simulink library "ee_lib_for_power_systems"  (R2025b)
settings: Solver=VariableStepAuto  StartTime=0.0  StopTime=10.0  RelTol=1e-3
undefined symbols (not defined in the file or in its folder): Alt_PolePair_Count, C3m, DiscIntNeverNeededParam, Enum1to12, L3m, Total_SM_Inertia, Ts
libraries: fl_lib x291, nesl_utility x219, ee_lib x139, custom_lib x12, $bdroot x10, ee_sl_lib x3, eeTransforms x2, eeGeneralControl x1, simulink x1
(part 3 of 11)

== system ee_lib_for_power_systems/Power Converters ==
  note: Lowest Fidelity
      | Use if switching not required.
      | Will short circuit AC if mod is 0.
  note: Three-Phase Rectifiers
  note: Other
  note: Three-Phase Inverters (simulation performance improves and fidelity decreases as you move from right to left)
  note: Individual Switches
  note: Link to Simscape Electrical Library
  note: Copyright (C) 2025, The MathWorks, Inc.
  note: Use if switching not required.
      | Rectifier mode if open is 1.
  note: Can accept subcycle average PWM.
      | Will short circuit AC if g is 0.
  note: Can accept subcycle average PWM.
      | Rectifier mode if open is 1.
  note: Use if switching required and diode forward voltage can be neglected.
      | Rectifier mode if open is 1.
  note: Highest Fidelity
      | Use if switching required and diode forward voltage cannot be neglected.
  note: Use for fastest thyristor bridge performance.
  note: Use for fastest half-bridge bridge performance.
  " Ideal Switch Three-Level NPC" : Subsystem  mask{Vf=1e-3, Ron=1e-3, Goff=1e-6, run_time=off}
      mask prompts: Vf "Forward Voltage (Vf)"; Ron "On Resistance (Ohm)"; Goff "Off Conductance (1/Ohm)"; run_time "Set parameters to run-time (model does not recompile if parameters change value)"
      mask Callback run_time:
      | run_time = get_param(gcb,'run_time');
      | 
      | path_converter = [gcb,'/converter'];
      | 
      | switch run_time
      |     case 'off'
      |         pcr = 'compiletime';
      |     case 'on'
      |         pcr = 'runtime';
      | end
      | 
      | set_param(path_converter,'Ron_conf',pcr)
      | set_param(path_converter,'Goff_conf',pcr)
      | set_param(path_converter,'Vf_conf',pcr)
      mask DialogControl DescGroupVar Prompt: Three-Level NPC Converter
      mask Display: disp('Ideal Switch\nThree-Level\nNPC')
  Average-Value VSC (Three-Phase) : Subsystem  mask{Ploss=1, run_time=off}
      mask prompts: Ploss "Fixed Power Loss (W)"; run_time "Set parameters to run-time (model does not recompile if parameters change value)"
      settings: PortSchema="{\"entries\":[{\"content\":{\"sides\":[{\"content\":{\"connectorIds\":[\"LConn1\"]},\"type\":\"ConnectorPlacement.EquallySpacedRectSide\",\"uuid\":\"86da145b-c877-4513-b106-d0dfb0b4d5fc\"},{\"content\":{\"connectorIds\":[\"RConn1\",\"RConn2\"],\"side\":\"RIGHT\"},\"type\":\"ConnectorPlacement.EquallySpacedRectSide\",\"uuid\":\"c4ddf9e5-f742-41c1-b9ac-c2cdd1e548bc\"},{\"content\":{\"connectorIds\":[\"In1\"],\"side\":\"TOP\"},\"type\":\"ConnectorPlacement.EquallySpacedRectSide\",\"uuid\":\"dcce36a4-1529-4432-9ca7-d054bd477a1d\"}]},\"type\":\"ConnectorPlacement.EquallySpacedConnector\",\"uuid\":\"4f4158e8-7d48-4204-9748-7ea34849688b\"}],\"packageUris\":[\"http://schema.mathworks.com/mf0/Connector/R2020b\"],\"version\":\"1.0\"}"
      mask Callback run_time:
      | run_time = get_param(gcb,'run_time');
      | 
      | path_converter = [gcb,'/converter'];
      | 
      | switch run_time
      |     case 'off'
      |         pcr = 'compiletime';
      |     case 'on'
      |         pcr = 'runtime';
      | end
      | 
      | set_param(path_converter,'Pfixed_conf',pcr)
      mask DialogControl Container1 Prompt: Average-Value VSC (Three-Phase)
      mask DialogControl Container2 Prompt: Parameters
      mask Display: disp('Average-Value\nVSC Bridge')
  Diode Bridge (Three-Phase) : Subsystem  mask{Vf=0.6, Ron=1e-3, Goff=1e-5}
      mask prompts: Vf "Forward Voltage (V)"; Ron "On Resistance (Ohm)"; Goff "Off Conductance"
      mask DialogControl DescGroupVar Prompt: Diode Bridge
      mask Display: disp('Diode\nBridge')
  GTO : Subsystem  mask{Ron=1e-5, Goff=1e-5, Vf=0.6, run_time=off}
      mask prompts: Ron "Ron (Ohm)"; Goff "Goff (1/Ohm)"; Vf "Vf (V)"; run_time "Set parameters to run-time (model does not recompile if parameters change value)"
      settings: PortSchema="{\"entries\":[{\"content\":{\"sides\":[{\"content\":{\"connectorIds\":[\"LConn1\"]},\"type\":\"ConnectorPlacement.EquallySpacedRectSide\",\"uuid\":\"886964a3-25ea-479f-932a-c2787c0416aa\"},{\"content\":{\"connectorIds\":[\"RConn1\"],\"side\":\"RIGHT\"},\"type\":\"ConnectorPlacement.EquallySpacedRectSide\",\"uuid\":\"91f12607-1466-497f-b2f1-8e90b455b295\"},{\"content\":{\"connectorIds\":[\"In1\"],\"side\":\"TOP\"},\"type\":\"ConnectorPlacement.EquallySpacedRectSide\",\"uuid\":\"4c118981-7ece-49ab-aa48-3dad47c140d6\"}]},\"type\":\"ConnectorPlacement.EquallySpacedConnector\",\"uuid\":\"0a5ac714-0cdd-4f48-b1a0-993e023eaa7d\"}],\"packageUris\":[\"http://schema.mathworks.com/mf0/Connector/R2020b\"],\"version\":\"1.0\"}"
      mask Callback run_time:
      | run_time = get_param(gcb,'run_time');
      | 
      | path_switch = [gcb,'/GTO'];
      | 
      | switch run_time
      |     case 'off'
      |         pcr = 'compiletime';
      |     case 'on'
      |         pcr = 'runtime';
      | end
      | 
      | set_param(path_switch,'Ron_conf',pcr)
      | set_param(path_switch,'Goff_conf',pcr)
      | set_param(path_switch,'Vf_conf',pcr)
      mask DialogControl DescGroupVar Prompt: GTO
      mask DialogControl Desc Prompt: Parameters
      mask Display: disp('GTO')
  GTO Bridge (Three-Phase) : Subsystem  mask{Ron=1e-5, Goff=1e-5, Vfs=1e-3, Vfd=1e-3, run_time=off}
      mask prompts: Ron "Ron (Ohm)"; Goff "Goff (1/Ohm)"; Vfs "GTO Forward Voltage (V)"; Vfd "Diode Forward Voltage (V)"; run_time "Set parameters to run-time (model does not recompile if parameters change value)"
      mask Callback run_time:
      | run_time = get_param(gcb,'run_time');
      | 
      | path_converter = [gcb,'/converter'];
      | 
      | switch run_time
      |     case 'off'
      |         pcr = 'compiletime';
      |     case 'on'
      |         pcr = 'runtime';
      | end
      | 
      | set_param(path_converter,'Ron_conf',pcr)
      | set_param(path_converter,'Goff_conf',pcr)
      | set_param(path_converter,'Vf_conf',pcr)
      | set_param(path_converter,'diode_Vf_conf',pcr)
      mask DialogControl DescGroupVar Prompt: GTO with Diode (Three-Phase)
      mask DialogControl DescTextVar Prompt: Gate inputs are natural commutation order.
      mask Display: disp('GTO\nBridge')
  IGBT : Subsystem  mask{Vf=0.6, Ron=1e-3, Goff=1e-5, run_time=off}
      mask prompts: Vf "Forward Voltage (V)"; Ron "On Resistance (Ohm)"; Goff "Off Conductance (1/Ohm)"; run_time "Set parameters to run-time (model does not recompile if parameters change value)"
      settings: PortSchema="{\"entries\":[{\"content\":{\"sides\":[{\"content\":{\"connectorIds\":[\"LConn1\"]},\"type\":\"ConnectorPlacement.EquallySpacedRectSide\",\"uuid\":\"cfcb5e16-4943-4b10-bb6e-0ab98dc97112\"},{\"content\":{\"connectorIds\":[\"RConn1\"],\"side\":\"RIGHT\"},\"type\":\"ConnectorPlacement.EquallySpacedRectSide\",\"uuid\":\"b5e3583a-b5f2-4cb8-a752-eb3fd656f5bf\"},{\"content\":{\"connectorIds\":[\"In1\"],\"side\":\"TOP\"},\"type\":\"ConnectorPlacement.EquallySpacedRectSide\",\"uuid\":\"dff9e97b-78f9-4e42-adbb-acb2cc9ce1e3\"}]},\"type\":\"ConnectorPlacement.EquallySpacedConnector\",\"uuid\":\"0da9f803-7999-4254-9390-1b84dddbfaeb\"}],\"packageUris\":[\"http://schema.mathworks.com/mf0/Connector/R2020b\"],\"version\":\"1.0\"}"
      mask Callback run_time:
      | run_time = get_param(gcb,'run_time');
      | 
      | path_switch = [gcb,'/IGBT'];
      | 
      | switch run_time
      |     case 'off'
      |         pcr = 'compiletime';
      |     case 'on'
      |         pcr = 'runtime';
      | end
      | 
      | set_param(path_switch,'Ron_conf',pcr)
      | set_param(path_switch,'Goff_conf',pcr)
      | set_param(path_switch,'Vf_conf',pcr)
      mask DialogControl DescGroupVar Prompt: IGBT
      mask Display: disp('IGBT')
  IGBT/Diode Bridge (Three-Phase) : Subsystem  mask{Ron=1e-5, Goff=1e-5, Vfs=1e-3, Vfd=1e-3, run_time=off}
      mask prompts: Ron "Ron (Ohm)"; Goff "Goff (1/Ohm)"; Vfs "IGBT Forward Voltage (V)"; Vfd "Diode Forward Voltage (V)"; run_time "Set parameters to run-time (model does not recompile if parameters change value)"
      mask Callback run_time:
      | run_time = get_param(gcb,'run_time');
      | 
      | path_converter = [gcb,'/converter'];
      | 
      | switch run_time
      |     case 'off'
      |         pcr = 'compiletime';
      |     case 'on'
      |         pcr = 'runtime';
      | end
      | 
      | set_param(path_converter,'Ron_conf',pcr)
      | set_param(path_converter,'Goff_conf',pcr)
      | set_param(path_converter,'Vf_conf',pcr)
      mask DialogControl DescGroupVar Prompt: IGBT/Diode Bridge (Three-Phase)
      mask Display: disp('IGBT/Diode\nBridge')
  Ideal Boost : Subsystem  mask{Vf=1e-3, Ron=1e-3, Goff=1e-6, L=1e-3, C=1e-6, run_time=on}
      mask prompts: Vf "Forward Voltage (Vf)"; Ron "On Resistance (Ohm)"; Goff "Off Conductance (1/Ohm)"; L "Inductance (H)"; C "Capacitance (F)"; run_time "Set parameters to run-time (model does not recompile if parameters change value)"
      mask Callback run_time:
      | run_time = get_param(gcb,'run_time');
      | 
      | path_boost = [gcb,'/boost'];
      | 
      | switch run_time
      |     case 'off'
      |         pcr = 'compiletime';
      |     case 'on'
      |         pcr = 'runtime';
      | end
      | 
      | set_param(path_boost,'Ron_conf',pcr)
      | set_param(path_boost,'Goff_conf',pcr)
      | set_param(path_boost,'Lf_conf',pcr)
      | set_param(path_boost,'Cf_conf',pcr)
      mask DialogControl DescGroupVar Prompt: Boost Converter
      mask Display: disp('Ideal\nBoost')
  Ideal Buck : Subsystem  mask{Vf=1e-3, Ron=1e-3, Goff=1e-6, L=1e-3, C=1e-6, run_time=on}
      mask prompts: Vf "Forward Voltage (Vf)"; Ron "On Resistance (Ohm)"; Goff "Off Conductance (1/Ohm)"; L "Inductance (H)"; C "Capacitance (F)"; run_time "Set parameters to run-time (model does not recompile if parameters change value)"
      mask Callback run_time:
      | run_time = get_param(gcb,'run_time');
      | 
      | path_boost = [gcb,'/buck'];
      | 
      | switch run_time
      |     case 'off'
      |         pcr = 'compiletime';
      |     case 'on'
      |         pcr = 'runtime';
      | end
      | 
      | set_param(path_boost,'Ron_conf',pcr)
      | set_param(path_boost,'Goff_conf',pcr)
      | set_param(path_boost,'Lf_conf',pcr)
      | set_param(path_boost,'Cf_conf',pcr)
      mask DialogControl DescGroupVar Prompt: Buck Converter
      mask Display: disp('Ideal\nBuck')
  Ideal Full-Bridge : Subsystem  mask{Vf=1e-3, Ron=1e-3, Goff=1e-6}
      mask prompts: Vf "Forward Voltage (Vf)"; Ron "On Resistance (Ohm)"; Goff "Off Conductance (1/Ohm)"
      mask DialogControl DescGroupVar Prompt: Full-Bridge Converter
      mask DialogControl DescTextVar Prompt: The full-bridge converter is modeled as four ideal switches with anti-parallel diodes.
      mask Display:
      | color('blue');
      | 
      | plot([0.2 0.7],[0.95 0.95])
      | plot([0.2 0.7],[0.05 0.05])
      | 
      | yoffset = 0.35;
      | 
      | %
      | plot([0.5 0.5],[0.9,0.7]-yoffset)
      | plot([0.5 0.5],[0.6 0.4]-yoffset)
      | plot([0.5 0.45],[0.6 0.7]-yoffset)
      | plot([0.4 0.5],[0.85 0.85]-yoffset)
      | 
      | 
      | %
      | plot(0.75,0.0)
      | plot(0,1)
      | 
      | % Diode
      | plot([0.5 0.6],[0.8 0.8]-yoffset)
      | plot([0.6 0.6],[0.8 0.7]-yoffset)
      | plot([0.6 0.55],[0.7 0.6]-yoffset)
      | plot([0.6 0.65],[0.7 0.6]-yoffset)
      | plot([0.55 0.65],[0.6 0.6]-yoffset)
      | plot([0.6 0.6],[0.5 0.6]-yoffset)
      | plot([0.5 0.6],[0.5 0.5]-yoffset)
      | plot([0.55 0.65],[0.7 0.7]-yoffset)
      | %
      | 
      | % upper
      | 
      | x1 = 0;
      | y1 = 0.4;
      | 
      | %
      | plot([0.5 0.5]+x1,[0.9,0.7]+y1-yoffset)
      | plot([0.5 0.5]+x1,[0.6 0.4]+y1-yoffset)
      | plot([0.5 0.45]+x1,[0.6 0.7]+y1-yoffset)
      | 
      | % Diode
      | plot([0.5 0.6]+x1,[0.8 0.8]+y1-yoffset)
      | plot([0.6 0.6]+x1,[0.8 0.7]+y1-yoffset)
      | plot([0.6 0.55]+x1,[0.7 0.6]+y1-yoffset)
      | plot([0.6 0.65]+x1,[0.7 0.6]+y1-yoffset)
      | plot([0.55 0.65]+x1,[0.6 0.6]+y1-yoffset)
      | plot([0.6 0.6]+x1,[0.5 0.6]+y1-yoffset)
      | plot([0.5 0.6]+x1,[0.5 0.5]+y1-yoffset)
      | plot([0.55 0.65]+x1,[0.7 0.7]+y1-yoffset)
      | %
      | 
      | %%%
      | 
      | xoffset = 0.3;
      | 
      | %
      | plot([0.5 0.5]-xoffset,[0.9,0.7]-yoffset)
      | plot([0.5 0.5]-xoffset,[0.6 0.4]-yoffset)
      | plot([0.5 0.45]-xoffset,[0.6 0.7]-yoffset)
      | plot([0.4 0.5]-xoffset,[0.85 0.85]-yoffset)
      | 
      | 
      | % Diode
      | plot([0.5 0.6]-xoffset,[0.8 0.8]-yoffset)
      | plot([0.6 0.6]-xoffset,[0.8 0.7]-yoffset)
      | plot([0.6 0.55]-xoffset,[0.7 0.6]-yoffset)
      | plot([0.6 0.65]-xoffset,[0.7 0.6]-yoffset)
      | plot([0.55 0.65]-xoffset,[0.6 0.6]-yoffset)
      | plot([0.6 0.6]-xoffset,[0.5 0.6]-yoffset)
      | plot([0.5 0.6]-xoffset,[0.5 0.5]-yoffset)
      | plot([0.55 0.65]-xoffset,[0.7 0.7]-yoffset)
      | %
      | 
      | % upper
      | 
      | x1 = 0;
      | y1 = 0.4;
      | 
      | %
      | plot([0.5 0.5]-xoffset+x1,[0.9,0.7]+y1-yoffset)
      | plot([0.5 0.5]-xoffset+x1,[0.6 0.4]+y1-yoffset)
      | plot([0.5 0.45]-xoffset+x1,[0.6 0.7]+y1-yoffset)
      | 
      | % Diode
      | plot([0.5 0.6]-xoffset+x1,[0.8 0.8]+y1-yoffset)
      | plot([0.6 0.6]-xoffset+x1,[0.8 0.7]+y1-yoffset)
      | plot([0.6 0.55]-xoffset+x1,[0.7 0.6]+y1-yoffset)
      | plot([0.6 0.65]-xoffset+x1,[0.7 0.6]+y1-yoffset)
      | plot([0.55 0.65]-xoffset+x1,[0.6 0.6]+y1-yoffset)
      | plot([0.6 0.6]-xoffset+x1,[0.5 0.6]+y1-yoffset)
      | plot([0.5 0.6]-xoffset+x1,[0.5 0.5]+y1-yoffset)
      | plot([0.55 0.65]-xoffset+x1,[0.7 0.7]+y1-yoffset)
      | %
  Ideal Half-Bridge : Subsystem  mask{Vf=1e-3, Ron=1e-3, Goff=1e-6, run_time=off}
      mask prompts: Vf "Forward Voltage (Vf)"; Ron "On Resistance (Ohm)"; Goff "Off Conductance (1/Ohm)"; run_time "Set parameters to run-time (model does not recompile if parameters change value)"
      mask Callback run_time:
      | run_time = get_param(gcb,'run_time');
      | 
      | path_S1 = [gcb,'/S1'];
      | path_S2 = [gcb,'/S2'];
      | path_D1 = [gcb,'/D1'];
      | path_D2 = [gcb,'/D2'];
      | 
      | switch run_time
      |     case 'off'
      |         pcr = 'compiletime';
      |     case 'on'
      |         pcr = 'runtime';
      | end
      | 
      | set_param(path_S1,'Ron_conf',pcr)
      | set_param(path_S1,'Goff_conf',pcr)
      | set_param(path_S2,'Ron_conf',pcr)
      | set_param(path_S2,'Goff_conf',pcr)
      | 
      | set_param(path_D1,'Ron_conf',pcr)
      | set_param(path_D1,'Goff_conf',pcr)
      | set_param(path_D1,'Vf_conf',pcr)
      | set_param(path_D2,'Ron_conf',pcr)
      | set_param(path_D2,'Goff_conf',pcr)
      | set_param(path_D2,'Vf_conf',pcr)
      mask DialogControl DescGroupVar Prompt: Half-Bridge Converter
      mask DialogControl DescTextVar Prompt: The half-bridge converter is modeled as two ideal switches with anti-parallel diodes.
      mask Display:
      | color('blue');
      | 
      | yoffset = 0.35;
      | 
      | %
      | plot([0.5 0.5],[0.9,0.7]-yoffset)
      | plot([0.5 0.5],[0.6 0.4]-yoffset)
      | plot([0.5 0.45],[0.6 0.7]-yoffset)
      | plot([0.4 0.5],[0.85 0.85]-yoffset)
      | 
      | 
      | %
      | plot(0.75,0.0)
      | plot(0.33,1)
      | 
      | % Diode
      | plot([0.5 0.6],[0.8 0.8]-yoffset)
      | plot([0.6 0.6],[0.8 0.7]-yoffset)
      | plot([0.6 0.55],[0.7 0.6]-yoffset)
      | plot([0.6 0.65],[0.7 0.6]-yoffset)
      | plot([0.55 0.65],[0.6 0.6]-yoffset)
      | plot([0.6 0.6],[0.5 0.6]-yoffset)
      | plot([0.5 0.6],[0.5 0.5]-yoffset)
      | plot([0.55 0.65],[0.7 0.7]-yoffset)
      | %
      | 
      | % upper
      | 
      | x1 = 0;
      | y1 = 0.4;
      | 
      | %
      | plot([0.5 0.5]+x1,[0.9,0.7]+y1-yoffset)
      | plot([0.5 0.5]+x1,[0.6 0.4]+y1-yoffset)
      | plot([0.5 0.45]+x1,[0.6 0.7]+y1-yoffset)
      | 
      | % Diode
      | plot([0.5 0.6]+x1,[0.8 0.8]+y1-yoffset)
      | plot([0.6 0.6]+x1,[0.8 0.7]+y1-yoffset)
      | plot([0.6 0.55]+x1,[0.7 0.6]+y1-yoffset)
      | plot([0.6 0.65]+x1,[0.7 0.6]+y1-yoffset)
      | plot([0.55 0.65]+x1,[0.6 0.6]+y1-yoffset)
      | plot([0.6 0.6]+x1,[0.5 0.6]+y1-yoffset)
      | plot([0.5 0.6]+x1,[0.5 0.5]+y1-yoffset)
      | plot([0.55 0.65]+x1,[0.7 0.7]+y1-yoffset)
      | %
  Ideal Half-Bridge (Basic Diode) : Subsystem  mask{Ron=1e-3, Goff=1e-6, run_time=off}
      mask prompts: Ron "On Resistance (Ohm)"; Goff "Off Conductance (1/Ohm)"; run_time "Set parameters to run-time (model does not recompile if parameters change value)"
      mask Callback run_time:
      | run_time = get_param(gcb,'run_time');
      | 
      | path_S1 = [gcb,'/S1'];
      | path_S2 = [gcb,'/S2'];
      | 
      | switch run_time
      |     case 'off'
      |         pcr = 'compiletime';
      |     case 'on'
      |         pcr = 'runtime';
      | end
      | 
      | set_param(path_S1,'Ron_conf',pcr)
      | set_param(path_S1,'Goff_conf',pcr)
      | set_param(path_S2,'Ron_conf',pcr)
      | set_param(path_S2,'Goff_conf',pcr)
      mask DialogControl DescGroupVar Prompt: Half-Bridge Converter
      mask DialogControl DescTextVar Prompt: The half-bridge converter is modeled as two basic switches with anti-parallel diodes. Diode forward voltage is fixed at 0V.
      mask Display:
      | color('blue');
      | 
      | yoffset = 0.35;
      | 
      | %
      | plot([0.5 0.5],[0.9,0.7]-yoffset)
      | plot([0.5 0.5],[0.6 0.4]-yoffset)
      | plot([0.5 0.45],[0.6 0.7]-yoffset)
      | plot([0.4 0.5],[0.85 0.85]-yoffset)
      | 
      | 
      | %
      | plot(0.75,0.0)
      | plot(0.33,1)
      | 
      | % Diode
      | plot([0.5 0.6],[0.8 0.8]-yoffset)
      | plot([0.6 0.6],[0.8 0.7]-yoffset)
      | plot([0.6 0.55],[0.7 0.6]-yoffset)
      | plot([0.6 0.65],[0.7 0.6]-yoffset)
      | plot([0.55 0.65],[0.6 0.6]-yoffset)
      | plot([0.6 0.6],[0.5 0.6]-yoffset)
      | plot([0.5 0.6],[0.5 0.5]-yoffset)
      | plot([0.55 0.65],[0.7 0.7]-yoffset)
      | %
      | 
      | % upper
      | 
      | x1 = 0;
      | y1 = 0.4;
      | 
      | %
      | plot([0.5 0.5]+x1,[0.9,0.7]+y1-yoffset)
      | plot([0.5 0.5]+x1,[0.6 0.4]+y1-yoffset)
      | plot([0.5 0.45]+x1,[0.6 0.7]+y1-yoffset)
      | 
      | % Diode
      | plot([0.5 0.6]+x1,[0.8 0.8]+y1-yoffset)
      | plot([0.6 0.6]+x1,[0.8 0.7]+y1-yoffset)
      | plot([0.6 0.55]+x1,[0.7 0.6]+y1-yoffset)
      | plot([0.6 0.65]+x1,[0.7 0.6]+y1-yoffset)
      | plot([0.55 0.65]+x1,[0.6 0.6]+y1-yoffset)
      | plot([0.6 0.6]+x1,[0.5 0.6]+y1-yoffset)
      | plot([0.5 0.6]+x1,[0.5 0.5]+y1-yoffset)
      | plot([0.55 0.65]+x1,[0.7 0.7]+y1-yoffset)
      | %
  Ideal Switch : Subsystem  mask{Ron=1e-3, Goff=1e-5, run_time=off}
      mask prompts: Ron "Ron (Ohm)"; Goff "Goff (1/Ohm)"; run_time "Set parameters to run-time (model does not recompile if parameters change value)"
      settings: PortSchema="{\"entries\":[{\"content\":{\"sides\":[{\"content\":{\"connectorIds\":[\"LConn1\"]},\"type\":\"ConnectorPlacement.EquallySpacedRectSide\",\"uuid\":\"886964a3-25ea-479f-932a-c2787c0416aa\"},{\"content\":{\"connectorIds\":[\"RConn1\"],\"side\":\"RIGHT\"},\"type\":\"ConnectorPlacement.EquallySpacedRectSide\",\"uuid\":\"91f12607-1466-497f-b2f1-8e90b455b295\"},{\"content\":{\"connectorIds\":[\"In1\"],\"side\":\"TOP\"},\"type\":\"ConnectorPlacement.EquallySpacedRectSide\",\"uuid\":\"4c118981-7ece-49ab-aa48-3dad47c140d6\"}]},\"type\":\"ConnectorPlacement.EquallySpacedConnector\",\"uuid\":\"0a5ac714-0cdd-4f48-b1a0-993e023eaa7d\"}],\"packageUris\":[\"http://schema.mathworks.com/mf0/Connector/R2020b\"],\"version\":\"1.0\"}"
      mask Callback run_time:
      | run_time = get_param(gcb,'run_time');
      | 
      | path_switch = [gcb,'/switch'];
      | 
      | switch run_time
      |     case 'off'
      |         pcr = 'compiletime';
      |     case 'on'
      |         pcr = 'runtime';
      | end
      | 
      | set_param(path_switch,'Ron_conf',pcr)
      | set_param(path_switch,'Goff_conf',pcr)
      mask DialogControl DescGroupVar Prompt: Ideal Switch
      mask Display: disp('Ideal Switch')
  Ideal Switch Basic Diode : Subsystem  mask{Ron=1e-3, Goff=1e-5, run_time=off}
      mask prompts: Ron "Ron (Ohm)"; Goff "Goff (1/Ohm)"; run_time "Set parameters to run-time (model does not recompile if parameters change value)"
      settings: PortSchema="{\"entries\":[{\"content\":{\"sides\":[{\"content\":{\"connectorIds\":[\"LConn1\"]},\"type\":\"ConnectorPlacement.EquallySpacedRectSide\",\"uuid\":\"886964a3-25ea-479f-932a-c2787c0416aa\"},{\"content\":{\"connectorIds\":[\"RConn1\"],\"side\":\"RIGHT\"},\"type\":\"ConnectorPlacement.EquallySpacedRectSide\",\"uuid\":\"91f12607-1466-497f-b2f1-8e90b455b295\"},{\"content\":{\"connectorIds\":[\"In1\"],\"side\":\"TOP\"},\"type\":\"ConnectorPlacement.EquallySpacedRectSide\",\"uuid\":\"4c118981-7ece-49ab-aa48-3dad47c140d6\"}]},\"type\":\"ConnectorPlacement.EquallySpacedConnector\",\"uuid\":\"0a5ac714-0cdd-4f48-b1a0-993e023eaa7d\"}],\"packageUris\":[\"http://schema.mathworks.com/mf0/Connector/R2020b\"],\"version\":\"1.0\"}"
      mask Callback run_time:
      | run_time = get_param(gcb,'run_time');
      | 
      | path_switch = [gcb,'/switch'];
      | 
      | switch run_time
      |     case 'off'
      |         pcr = 'compiletime';
      |     case 'on'
      |         pcr = 'runtime';
      | end
      | 
      | set_param(path_switch,'Ron_conf',pcr)
      | set_param(path_switch,'Goff_conf',pcr)
      mask DialogControl DescGroupVar Prompt: Ideal Switch Basic Diode
      mask DialogControl DescTextVar Prompt: Ideal switch with anti-parallel diode. Forward voltage is fixed at 0V.
      mask Display: disp('Ideal Switch\nBasic Diode')
  Ideal Switch Basic Diode Two-Level : Subsystem  mask{Ron=1e-3, Goff=1e-6}
      mask prompts: Ron "On Resistance (Ohm)"; Goff "Off Conductance (1/Ohm)"
      settings: PortSchema="{\"entries\":[{\"content\":{\"sides\":[{\"content\":{\"connectorIds\":[\"In1\",\"LConn1\"]},\"type\":\"ConnectorPlacement.EquallySpacedRectSide\",\"uuid\":\"0a5abffb-b862-46b7-a047-f0c05c2594f4\"},{\"content\":{\"connectorIds\":[\"RConn1\",\"RConn2\"],\"side\":\"RIGHT\"},\"type\":\"ConnectorPlacement.EquallySpacedRectSide\",\"uuid\":\"46c33ab7-91d4-4fb9-a847-19c4ea881524\"},{\"content\":{\"connectorIds\":[\"In2\"],\"side\":\"TOP\"},\"type\":\"ConnectorPlacement.EquallySpacedRectSide\",\"uuid\":\"65680121-e081-4085-a2db-0359a3903d83\"}]},\"type\":\"ConnectorPlacement.EquallySpacedConnector\",\"uuid\":\"d4058c4e-3a99-4efe-af9f-90087adb625e\"}],\"packageUris\":[\"http://schema.mathworks.com/mf0/Connector/R2020b\"],\"version\":\"1.0\"}"
      mask DialogControl DescGroupVar Prompt: Ideal Switch Basic Diode Two-Level Converter
      mask DialogControl DescTextVar Prompt: Ideal switch characterized by Ron and Roff. Diode forward voltage fixed at 0V.
      mask Display: disp('Ideal Switch\nBasic Diode\nTwo-Level')
  Ideal Switch Two-Level : Subsystem  mask{Vf=1e-3, Ron=1e-3, Goff=1e-6, run_time=off}
      mask prompts: Vf "Forward Voltage (Vf)"; Ron "On Resistance (Ohm)"; Goff "Off Conductance (1/Ohm)"; run_time "Set parameters to run-time (model does not recompile if parameters change value)"
      mask Callback run_time:
      | run_time = get_param(gcb,'run_time');
      | 
      | path_converter = [gcb,'/converter'];
      | 
      | switch run_time
      |     case 'off'
      |         pcr = 'compiletime';
      |     case 'on'
      |         pcr = 'runtime';
      | end
      | 
      | set_param(path_converter,'Ron_conf',pcr)
      | set_param(path_converter,'Goff_conf',pcr)
      | set_param(path_converter,'Vf_conf',pcr)
      mask DialogControl DescGroupVar Prompt: Two-Level Converter
      mask Display: disp('Ideal Switch\nTwo-Level')
  MOSFET : Subsystem  mask{Ron=1e-3, Goff=1e-5, run_time=off}
      mask prompts: Ron "On Resistance (Ohm)"; Goff "Off Conductance (1/Ohm)"; run_time "Set parameters to run-time (model does not recompile if parameters change value)"
      settings: PortSchema="{\"entries\":[{\"content\":{\"sides\":[{\"content\":{\"connectorIds\":[\"LConn1\"]},\"type\":\"ConnectorPlacement.EquallySpacedRectSide\",\"uuid\":\"886964a3-25ea-479f-932a-c2787c0416aa\"},{\"content\":{\"connectorIds\":[\"RConn1\"],\"side\":\"RIGHT\"},\"type\":\"ConnectorPlacement.EquallySpacedRectSide\",\"uuid\":\"91f12607-1466-497f-b2f1-8e90b455b295\"},{\"content\":{\"connectorIds\":[\"In1\"],\"side\":\"TOP\"},\"type\":\"ConnectorPlacement.EquallySpacedRectSide\",\"uuid\":\"4c118981-7ece-49ab-aa48-3dad47c140d6\"}]},\"type\":\"ConnectorPlacement.EquallySpacedConnector\",\"uuid\":\"0a5ac714-0cdd-4f48-b1a0-993e023eaa7d\"}],\"packageUris\":[\"http://schema.mathworks.com/mf0/Connector/R2020b\"],\"version\":\"1.0\"}"
      mask Callback run_time:
      | run_time = get_param(gcb,'run_time');
      | 
      | path_switch = [gcb,'/MOSFET'];
      | 
      | switch run_time
      |     case 'off'
      |         pcr = 'compiletime';
      |     case 'on'
      |         pcr = 'runtime';
      | end
      | 
      | set_param(path_switch,'Rds_conf',pcr)
      | set_param(path_switch,'Goff_conf',pcr)
      mask DialogControl DescGroupVar Prompt: MOSFET
      mask Display: disp('MOSFET')
  MOSFET/Diode Bridge (Three-Phase) : Subsystem  mask{Ron=1e-5, Goff=1e-5, Vfd=1e-3, run_time=off}
      mask prompts: Ron "Ron (Ohm)"; Goff "Goff (1/Ohm)"; Vfd "Diode Forward Voltage (V)"; run_time "Set parameters to run-time (model does not recompile if parameters change value)"
      mask Callback run_time:
      | run_time = get_param(gcb,'run_time');
      | 
      | path_converter = [gcb,'/converter'];
      | 
      | switch run_time
      |     case 'off'
      |         pcr = 'compiletime';
      |     case 'on'
      |         pcr = 'runtime';
      | end
      | 
      | set_param(path_converter,'Ron_conf',pcr)
      | set_param(path_converter,'Goff_conf',pcr)
      mask DialogControl DescGroupVar Prompt: MOSFET/Diode Bridge (Three-Phase)
      mask Display: disp('MOSFET/Diode\nBridge')
  Semiconductors & Converters : ee_lib/Semiconductors & Converters  Tag=simscape_sublibrary  SystemSampleTime=-1  VariantActivationTime=update diagram  EmptyChoice=off  PassThrough=off  PassThroughVariantControl=(default)  EmptyChoiceVariantControl=(default)  AllowFlexibleInterface=on  Latency=0  AutoFrameSizeCalculation=off
  Three-Level Average-Value : Subsystem  mask{Vf=1e-3, Ron=1e-5, Goff=1e-5}
      mask prompts: Vf "Diode Forward Voltage (V)"; Ron "Diode On Resistance (Ohm)"; Goff "Diode Off Conductance (1/Ohm)"
      settings: PortSchema="{\"entries\":[{\"content\":{\"sides\":[{\"content\":{\"connectorIds\":[\"LConn1\",\"LConn2\",\"LConn3\"]},\"type\":\"ConnectorPlacement.EquallySpacedRectSide\",\"uuid\":\"e78ecab8-65b9-483d-b75b-425babe0c3e1\"},{\"content\":{\"connectorIds\":[\"In2\",\"RConn1\"],\"side\":\"RIGHT\"},\"type\":\"ConnectorPlacement.EquallySpacedRectSide\",\"uuid\":\"a274fc82-541f-4f7c-b01e-96e9d6625cc4\"},{\"content\":{\"connectorIds\":[\"In1\"],\"side\":\"TOP\"},\"type\":\"ConnectorPlacement.EquallySpacedRectSide\",\"uuid\":\"7f1df5be-1465-4e93-8e59-22e6d60c16e3\"}]},\"type\":\"ConnectorPlacement.EquallySpacedConnector\",\"uuid\":\"b266f7d3-0ec9-40d4-9eef-74ce8af397f6\"}],\"packageUris\":[\"http://schema.mathworks.com/mf0/Connector/R2020b\"],\"version\":\"1.0\"}"
      mask DialogControl DescGroupVar Prompt: Three-Level Average-Value
      mask DialogControl DescTextVar Prompt: Models a three-level NPC as average value with diodes. Set 'open' to 1 to configure as a diode bridge.
      mask Display: disp('Three-Level\nAverage-Value\n(Ineut = 0)')
  Three-Level NPC Average-Switch with Diodes : Subsystem  mask{Vf=1e-4, Ron=1e-4, Goff=1e-4, Ts=5e-5}
      mask prompts: Vf "Diode Forward Voltage (V)"; Ron "Diode On Resistance (Ohm)"; Goff "Diode Off Conductance (1/Ohm)"; Ts "Sample Time (s)"
      settings: PortSchema="{\"entries\":[{\"content\":{\"sides\":[{\"content\":{\"connectorIds\":[\"LConn1\",\"LConn2\",\"LConn3\"]},\"type\":\"ConnectorPlacement.EquallySpacedRectSide\",\"uuid\":\"e78ecab8-65b9-483d-b75b-425babe0c3e1\"},{\"content\":{\"connectorIds\":[\"In2\",\"RConn1\"],\"side\":\"RIGHT\"},\"type\":\"ConnectorPlacement.EquallySpacedRectSide\",\"uuid\":\"a274fc82-541f-4f7c-b01e-96e9d6625cc4\"},{\"content\":{\"connectorIds\":[\"In1\"],\"side\":\"TOP\"},\"type\":\"ConnectorPlacement.EquallySpacedRectSide\",\"uuid\":\"7f1df5be-1465-4e93-8e59-22e6d60c16e3\"}]},\"type\":\"ConnectorPlacement.EquallySpacedConnector\",\"uuid\":\"b266f7d3-0ec9-40d4-9eef-74ce8af397f6\"}],\"packageUris\":[\"http://schema.mathworks.com/mf0/Connector/R2020b\"],\"version\":\"1.0\"}"
      mask DialogControl DescGroupVar Prompt: Three-Level NPC Average-Switch (with Diodes)
      mask DialogControl DescTextVar Prompt: Accepts PWM or averaged PWM signals. Acts as a diode rectifier if open is set to 1.
      mask Display: disp('Three-Level\nNPC\nAverage-Switch\nwith Diodes')
  Thyristor : Subsystem  mask{Ron=1e-5, Goff=1e-5, Vf=0.6, run_time=off}
      mask prompts: Ron "Ron (Ohm)"; Goff "Goff (1/Ohm)"; Vf "Vf (V)"; run_time "Set parameters to run-time (model does not recompile if parameters change value)"
      settings: PortSchema="{\"entries\":[{\"content\":{\"sides\":[{\"content\":{\"connectorIds\":[\"LConn1\"]},\"type\":\"ConnectorPlacement.EquallySpacedRectSide\",\"uuid\":\"886964a3-25ea-479f-932a-c2787c0416aa\"},{\"content\":{\"connectorIds\":[\"RConn1\"],\"side\":\"RIGHT\"},\"type\":\"ConnectorPlacement.EquallySpacedRectSide\",\"uuid\":\"91f12607-1466-497f-b2f1-8e90b455b295\"},{\"content\":{\"connectorIds\":[\"In1\"],\"side\":\"TOP\"},\"type\":\"ConnectorPlacement.EquallySpacedRectSide\",\"uuid\":\"4c118981-7ece-49ab-aa48-3dad47c140d6\"}]},\"type\":\"ConnectorPlacement.EquallySpacedConnector\",\"uuid\":\"0a5ac714-0cdd-4f48-b1a0-993e023eaa7d\"}],\"packageUris\":[\"http://schema.mathworks.com/mf0/Connector/R2020b\"],\"version\":\"1.0\"}"
      mask Callback run_time:
      | run_time = get_param(gcb,'run_time');
      | 
      | path_switch = [gcb,'/thyristor'];
      | 
      | switch run_time
      |     case 'off'
      |         pcr = 'compiletime';
      |     case 'on'
      |         pcr = 'runtime';
      | end
      | 
      | set_param(path_switch,'Ron_conf',pcr)
      | set_param(path_switch,'Goff_conf',pcr)
      | set_param(path_switch,'Vf_conf',pcr)
      mask DialogControl DescGroupVar Prompt: Thyristor
      mask Display: disp('Thyristor')
  Thyristor Bridge (Ideal) : Subsystem  mask{Ron=1e-5, Goff=1e-5, Vf=1e-3}
      mask prompts: Ron "Ron (Ohm)"; Goff "Goff (1/Ohm)"; Vf "Vf (V)"
      mask DialogControl DescGroupVar Prompt: Ideal Thyristor
      mask DialogControl DescTextVar Prompt: Gate inputs are natural commutation order.
      mask Display: disp('Thyristor\nBridge\n(Ideal)')
  Thyristor Bridge (Three-Phase) : Subsystem  mask{Ron=1e-5, Goff=1e-5, Vfs=1e-3, run_time=off}
      mask prompts: Ron "Ron (Ohm)"; Goff "Goff (1/Ohm)"; Vfs "Forward Voltage (V)"; run_time "Set parameters to run-time (model does not recompile if parameters change value)"
      mask Callback run_time:
      | run_time = get_param(gcb,'run_time');
      | 
      | path_converter = [gcb,'/converter'];
      | 
      | switch run_time
      |     case 'off'
      |         pcr = 'compiletime';
      |     case 'on'
      |         pcr = 'runtime';
      | end
      | 
      | set_param(path_converter,'Ron_conf',pcr)
      | set_param(path_converter,'Goff_conf',pcr)
      | set_param(path_converter,'Vf_conf',pcr)
      mask DialogControl DescGroupVar Prompt: Thyristor Bridge (Three-Phase)
      mask DialogControl DescTextVar Prompt: Gate inputs are natural commutation order.
      mask Display: disp('Thyristor\nBridge')
  Two-Level Average-Switch : Subsystem
      settings: PortSchema="{\"entries\":[{\"content\":{\"sides\":[{\"content\":{\"connectorIds\":[\"LConn1\",\"LConn2\"]},\"type\":\"ConnectorPlacement.EquallySpacedRectSide\",\"uuid\":\"e78ecab8-65b9-483d-b75b-425babe0c3e1\"},{\"content\":{\"connectorIds\":[\"In1\",\"RConn1\"],\"side\":\"RIGHT\"},\"type\":\"ConnectorPlacement.EquallySpacedRectSide\",\"uuid\":\"a274fc82-541f-4f7c-b01e-96e9d6625cc4\"},{\"content\":{\"side\":\"TOP\"},\"type\":\"ConnectorPlacement.EquallySpacedRectSide\",\"uuid\":\"7f1df5be-1465-4e93-8e59-22e6d60c16e3\"}]},\"type\":\"ConnectorPlacement.EquallySpacedConnector\",\"uuid\":\"b266f7d3-0ec9-40d4-9eef-74ce8af397f6\"}],\"packageUris\":[\"http://schema.mathworks.com/mf0/Connector/R2020b\"],\"version\":\"1.0\"}"
      mask Display: disp('Two-Level\nAverage Switch\n(No Diodes)')
  Two-Level Average-Switch with Diodes : Subsystem  mask{Vf=1e-3, Ron=1e-5, Goff=1e-5}
      mask prompts: Vf "Diode Forward Voltage (V)"; Ron "Diode On Resistance (Ohm)"; Goff "Diode Off Conductance (1/Ohm)"
      settings: PortSchema="{\"entries\":[{\"content\":{\"sides\":[{\"content\":{\"connectorIds\":[\"LConn1\",\"LConn2\"]},\"type\":\"ConnectorPlacement.EquallySpacedRectSide\",\"uuid\":\"e78ecab8-65b9-483d-b75b-425babe0c3e1\"},{\"content\":{\"connectorIds\":[\"In2\",\"RConn1\"],\"side\":\"RIGHT\"},\"type\":\"ConnectorPlacement.EquallySpacedRectSide\",\"uuid\":\"a274fc82-541f-4f7c-b01e-96e9d6625cc4\"},{\"content\":{\"connectorIds\":[\"In1\"],\"side\":\"TOP\"},\"type\":\"ConnectorPlacement.EquallySpacedRectSide\",\"uuid\":\"7f1df5be-1465-4e93-8e59-22e6d60c16e3\"}]},\"type\":\"ConnectorPlacement.EquallySpacedConnector\",\"uuid\":\"b266f7d3-0ec9-40d4-9eef-74ce8af397f6\"}],\"packageUris\":[\"http://schema.mathworks.com/mf0/Connector/R2020b\"],\"version\":\"1.0\"}"
      mask DialogControl DescGroupVar Prompt: Two-Level Average Switch with Diodes
      mask DialogControl DescTextVar Prompt: Accepts PWM signals and models controllable switches as voltage sources. To open all switches, set 'open' to 1.
      mask Display: disp('Two-Level\nAverage Switch\nWith Diodes')
  Two-Level Average-Value : Subsystem  mask{Vf=1e-3, Ron=1e-5, Goff=1e-5}
      mask prompts: Vf "Diode Forward Voltage (V)"; Ron "Diode On Resistance (Ohm)"; Goff "Diode Off Conductance (1/Ohm)"
      settings: PortSchema="{\"entries\":[{\"content\":{\"sides\":[{\"content\":{\"connectorIds\":[\"LConn1\",\"LConn2\"]},\"type\":\"ConnectorPlacement.EquallySpacedRectSide\",\"uuid\":\"e78ecab8-65b9-483d-b75b-425babe0c3e1\"},{\"content\":{\"connectorIds\":[\"In2\",\"RConn1\"],\"side\":\"RIGHT\"},\"type\":\"ConnectorPlacement.EquallySpacedRectSide\",\"uuid\":\"a274fc82-541f-4f7c-b01e-96e9d6625cc4\"},{\"content\":{\"connectorIds\":[\"In1\"],\"side\":\"TOP\"},\"type\":\"ConnectorPlacement.EquallySpacedRectSide\",\"uuid\":\"7f1df5be-1465-4e93-8e59-22e6d60c16e3\"}]},\"type\":\"ConnectorPlacement.EquallySpacedConnector\",\"uuid\":\"b266f7d3-0ec9-40d4-9eef-74ce8af397f6\"}],\"packageUris\":[\"http://schema.mathworks.com/mf0/Connector/R2020b\"],\"version\":\"1.0\"}"
      mask DialogControl DescGroupVar Prompt: Three-Level Average-Value
      mask DialogControl DescTextVar Prompt: Models a three-level NPC as average value with diodes. Set 'open' to 1 to configure as a diode bridge.
      mask Display: disp('Two-Level\nAverage-Value\nwith Diodes')

== system ee_lib_for_power_systems/Sense and Measure ==
  note: Fourier analysis and filters in here ----------->
  note: Links to Simscape Electrical Libraries
  note: Copyright (C) 2025, The MathWorks, Inc.
  note: PLLs, sequence analyzer, THD, RMS, power measurement in here ----------->
  General Control : ee_sl_lib/General Control  SystemSampleTime=-1  VariantActivationTime=update diagram  EmptyChoice=off  PassThrough=off  PassThroughVariantControl=(default)  EmptyChoiceVariantControl=(default)  AllowFlexibleInterface=on  Latency=0  AutoFrameSizeCalculation=off
  Measurements : ee_sl_lib/Measurements  SystemSampleTime=-1  VariantActivationTime=update diagram  EmptyChoice=off  PassThrough=off  PassThroughVariantControl=(default)  EmptyChoiceVariantControl=(default)  AllowFlexibleInterface=on  Latency=0  AutoFrameSizeCalculation=off
  Power sensor (Three-Phase) : Subsystem
      settings: PortSchema="{\"entries\":[{\"content\":{\"sides\":[{\"content\":{\"connectorIds\":[\"LConn1\"]},\"type\":\"ConnectorPlacement.EquallySpacedRectSide\",\"uuid\":\"d536c57f-d596-4c30-b2f2-ca7a7128ca09\"},{\"content\":{\"connectorIds\":[\"RConn1\"],\"side\":\"RIGHT\"},\"type\":\"ConnectorPlacement.EquallySpacedRectSide\",\"uuid\":\"3e38bcb4-aad6-4c8e-8ba0-f34fad0d941d\"},{\"content\":{\"connectorIds\":[\"Out1\",\"Out2\"],\"side\":\"TOP\"},\"type\":\"ConnectorPlacement.EquallySpacedRectSide\",\"uuid\":\"dbbb5dbf-676f-46fa-997f-a90b77759865\"}]},\"type\":\"ConnectorPlacement.EquallySpacedConnector\",\"uuid\":\"d250c4d3-27c8-4176-913c-392fad876444\"}],\"packageUris\":[\"http://schema.mathworks.com/mf0/Connector/R2020b\"],\"version\":\"1.0\"}"
      mask Display: disp('PQ')
  Sensors & Transducers : ee_lib/Sensors & Transducers  Tag=simscape_sublibrary  SystemSampleTime=-1  VariantActivationTime=update diagram  EmptyChoice=off  PassThrough=off  PassThroughVariantControl=(default)  EmptyChoiceVariantControl=(default)  AllowFlexibleInterface=on  Latency=0  AutoFrameSizeCalculation=off
  VI sensor (Three-Phase) : Subsystem  mask{volt_meas=Phase-to-Ground (Phase Voltage), meas_units=SI, Vnom=24e3, Pnom=100e6}
      mask prompts: volt_meas "Voltage Measurement"; meas_units "Measurement Units"; Vnom "Nominal Voltage (Vrms)"; Pnom "Nominal Power (VA)"
      mask init:
      | volt_meas  = get_param(gcb,'volt_meas');
      | meas_units = get_param(gcb,'meas_units');
      | 
      | path_CV = [gcb,'/current_voltage'];
      | 
      | switch volt_meas
      | 
      |     case 'Phase-to-Ground (Phase Voltage)'
      | 
      |         set_param(path_CV,'vMeasurementType','ee.enum.vmeasurement.lg')
      | 
      |     case 'Line-to-Line (Line Voltage)'
      | 
      |         set_param(path_CV,'vMeasurementType','ee.enum.vmeasurement.ll')
      | 
      | end
      | 
      | switch meas_units
      | 
      |     case 'SI'
      | 
      |         set_param(path_CV,'outputUnit','ee.enum.unit.si')
      |         
      | 
      |     case 'PU'
      | 
      |         set_param(path_CV,'outputUnit','ee.enum.unit.pu')
      |       
      | 
      | end
      settings: PortSchema="{\"entries\":[{\"content\":{\"sides\":[{\"content\":{\"connectorIds\":[\"LConn1\"]},\"type\":\"ConnectorPlacement.EquallySpacedRectSide\",\"uuid\":\"66880061-d275-464c-ab2c-563a1dd2cd8b\"},{\"content\":{\"connectorIds\":[\"RConn1\"],\"side\":\"RIGHT\"},\"type\":\"ConnectorPlacement.EquallySpacedRectSide\",\"uuid\":\"61b04713-3835-4ea6-b554-2bb2ace28c8c\"},{\"content\":{\"connectorIds\":[\"Out1\",\"Out2\"],\"side\":\"TOP\"},\"type\":\"ConnectorPlacement.EquallySpacedRectSide\",\"uuid\":\"be795a7c-014c-44ea-ab59-513af0394dbb\"}]},\"type\":\"ConnectorPlacement.EquallySpacedConnector\",\"uuid\":\"739e21ea-20b0-4a9e-9950-77186c3d44aa\"}],\"packageUris\":[\"http://schema.mathworks.com/mf0/Connector/R2020b\"],\"version\":\"1.0\"}"
      mask TypeOptions volt_meas: Phase-to-Ground (Phase Voltage) | Line-to-Line (Line Voltage)
      mask TypeOptions meas_units: SI | PU
      mask Callback meas_units:
      | meas_units = get_param(gcb,'meas_units');
      | 
      | switch meas_units
      | 
      |     case 'SI'
      | 
      |         set_param(gcb,'MaskVisibilities',{'on','on','off','off'})
      | 
      | 
      |     case 'PU'
      | 
      |         set_param(gcb,'MaskVisibilities',{'on','on','on','on'})
      | 
      | 
      | end
      mask DialogControl Container1 Prompt: Voltage and Current Measurement
      mask DialogControl Container2 Prompt: The block represents an ideal three-phase voltage and current sensor.
  Vectorized PQ meas : Subsystem  DialogController=POWERSYS.PowerSysDialog
      settings: LibrarySourceBlock=|
      | sps_lib/Sensors and Measurements/Power
      | (3ph, Instantaneous)
  current sensor : Subsystem
      settings: PortSchema="{\"entries\":[{\"content\":{\"sides\":[{\"content\":{\"connectorIds\":[\"LConn1\"]},\"type\":\"ConnectorPlacement.EquallySpacedRectSide\",\"uuid\":\"799648ba-196f-427a-9565-f64fffdae700\"},{\"content\":{\"connectorIds\":[\"RConn1\"],\"side\":\"RIGHT\"},\"type\":\"ConnectorPlacement.EquallySpacedRectSide\",\"uuid\":\"662de5d5-c2ef-406c-9235-94a35f9d89fb\"},{\"content\":{\"connectorIds\":[\"Out1\"],\"side\":\"TOP\"},\"type\":\"ConnectorPlacement.EquallySpacedRectSide\",\"uuid\":\"67dc8fec-98bd-426f-bbe6-5723f2587b12\"}]},\"type\":\"ConnectorPlacement.EquallySpacedConnector\",\"uuid\":\"3e33dbb3-529a-4788-9727-18d62c402281\"}],\"packageUris\":[\"http://schema.mathworks.com/mf0/Connector/R2020b\"],\"version\":\"1.0\"}"
  multi-phase current sensor : SimscapeComponentBlock  SourceFile=multi_current  ComponentPath=multi_current  ComponentVariants="[\"multi_current\"]"  ComponentVariantNames="[\"multi_current\"]"  ClassName=multi_current  SchemaVersion=1  noph=3
      settings: CopyFcn=simscape.sscblock.internal.componentBlockCallback(gcbh,'COPY')  PreCopyFcn=simscape.sscblock.internal.componentBlockCallback(gcbh,'PRECOPY')  PreDeleteFcn=simscape.sscblock.internal.componentBlockCallback(gcbh,'PREDELETE')  InternalSimscapePortConfiguration="{\"Bottom\":[],\"Left\":[{\"id\":\"p\",\"label\":\"+\",\"type\":\"foundation.electrical.electrical\"}],\"Right\":[{\"id\":\"I\",\"label\":\"I\",\"type\":\"output\"},{\"id\":\"n\",\"label\":\"-\",\"type\":\"foundation.electrical.electrical\"}],\"Top\":[]}"
      mask Type:
      | multi-phase
      | current sensor
  multi-phase voltage sensor : SimscapeComponentBlock  SourceFile=multi_voltage  ComponentPath=multi_voltage  ComponentVariants="[\"multi_voltage\"]"  ComponentVariantNames="[\"multi_voltage\"]"  ClassName=multi_voltage  SchemaVersion=1  noph=3
      settings: CopyFcn=simscape.sscblock.internal.componentBlockCallback(gcbh,'COPY')  PreCopyFcn=simscape.sscblock.internal.componentBlockCallback(gcbh,'PRECOPY')  PreDeleteFcn=simscape.sscblock.internal.componentBlockCallback(gcbh,'PREDELETE')  InternalSimscapePortConfiguration="{\"Bottom\":[],\"Left\":[{\"id\":\"p\",\"label\":\"+\",\"type\":\"foundation.electrical.electrical\"}],\"Right\":[{\"id\":\"V\",\"label\":\"V\",\"type\":\"output\"},{\"id\":\"n\",\"label\":\"-\",\"type\":\"foundation.electrical.electrical\"}],\"Top\":[]}"
      mask Type:
      | multi-phase
      | voltage sensor
  voltage sensor : Subsystem
      settings: PortSchema="{\"entries\":[{\"content\":{\"sides\":[{\"content\":{},\"type\":\"ConnectorPlacement.EquallySpacedRectSide\",\"uuid\":\"799648ba-196f-427a-9565-f64fffdae700\"},{\"content\":{\"connectorIds\":[\"Out1\"],\"side\":\"RIGHT\"},\"type\":\"ConnectorPlacement.EquallySpacedRectSide\",\"uuid\":\"662de5d5-c2ef-406c-9235-94a35f9d89fb\"},{\"content\":{\"connectorIds\":[\"LConn1\"],\"side\":\"TOP\"},\"type\":\"ConnectorPlacement.EquallySpacedRectSide\",\"uuid\":\"67dc8fec-98bd-426f-bbe6-5723f2587b12\"},{\"content\":{\"connectorIds\":[\"RConn1\"],\"side\":\"BOTTOM\"},\"type\":\"ConnectorPlacement.EquallySpacedRectSide\",\"uuid\":\"7e3d7354-1ab6-44d8-9b44-c988118d052c\"}]},\"type\":\"ConnectorPlacement.EquallySpacedConnector\",\"uuid\":\"3e33dbb3-529a-4788-9727-18d62c402281\"}],\"packageUris\":[\"http://schema.mathworks.com/mf0/Connector/R2020b\"],\"version\":\"1.0\"}"

== system ee_lib_for_power_systems/Sources ==
  note: Link to Simscape Electrical library
  note: Copyright (C) 2025, The MathWorks, Inc.
  Controlled Current Source : Subsystem
      settings: PortSchema="{\"entries\":[{\"content\":{\"sides\":[{\"content\":{\"connectorIds\":[\"In1\"]},\"type\":\"ConnectorPlacement.EquallySpacedRectSide\",\"uuid\":\"090b2ee3-4378-4784-ad56-1af8514f6806\"},{\"content\":{\"side\":\"RIGHT\"},\"type\":\"ConnectorPlacement.EquallySpacedRectSide\",\"uuid\":\"4adbd240-d61e-4082-b4ef-02f0dbd361b1\"},{\"content\":{\"connectorIds\":[\"LConn1\"],\"side\":\"TOP\"},\"type\":\"ConnectorPlacement.EquallySpacedRectSide\",\"uuid\":\"34d099db-9580-46ff-aa35-d8c98d9402ef\"},{\"content\":{\"connectorIds\":[\"RConn1\"],\"side\":\"BOTTOM\"},\"type\":\"ConnectorPlacement.EquallySpacedRectSide\",\"uuid\":\"52aceab2-4099-4b55-8f08-47c5423f9985\"}]},\"type\":\"ConnectorPlacement.EquallySpacedConnector\",\"uuid\":\"cb3dc6bb-ab47-4bf2-a22f-b68fe84c97d5\"}],\"packageUris\":[\"http://schema.mathworks.com/mf0/Connector/R2020b\"],\"version\":\"1.0\"}"
  Controlled Voltage Source : Subsystem
      settings: PortSchema="{\"entries\":[{\"content\":{\"sides\":[{\"content\":{\"connectorIds\":[\"In1\"]},\"type\":\"ConnectorPlacement.EquallySpacedRectSide\",\"uuid\":\"090b2ee3-4378-4784-ad56-1af8514f6806\"},{\"content\":{\"side\":\"RIGHT\"},\"type\":\"ConnectorPlacement.EquallySpacedRectSide\",\"uuid\":\"4adbd240-d61e-4082-b4ef-02f0dbd361b1\"},{\"content\":{\"connectorIds\":[\"LConn1\"],\"side\":\"TOP\"},\"type\":\"ConnectorPlacement.EquallySpacedRectSide\",\"uuid\":\"34d099db-9580-46ff-aa35-d8c98d9402ef\"},{\"content\":{\"connectorIds\":[\"RConn1\"],\"side\":\"BOTTOM\"},\"type\":\"ConnectorPlacement.EquallySpacedRectSide\",\"uuid\":\"ff207868-e9a4-4c5d-8c6f-607ccff15d02\"}]},\"type\":\"ConnectorPlacement.EquallySpacedConnector\",\"uuid\":\"cb3dc6bb-ab47-4bf2-a22f-b68fe84c97d5\"}],\"packageUris\":[\"http://schema.mathworks.com/mf0/Connector/R2020b\"],\"version\":\"1.0\"}"
  Fuel Cell : Subsystem
  Solar Array : Subsystem
  Sources : ee_lib/Sources  Tag=simscape_sublibrary  SystemSampleTime=-1  VariantActivationTime=update diagram  EmptyChoice=off  PassThrough=off  PassThroughVariantControl=(default)  EmptyChoiceVariantControl=(default)  AllowFlexibleInterface=on  Latency=0  AutoFrameSizeCalculation=off

== system ee_lib_for_power_systems/Transformations ==
  note: Link to Simscape Electrical library
  note: Copyright (C) 2025, The MathWorks, Inc.
  Mathematical Transforms : ee_sl_lib/Mathematical Transforms  SystemSampleTime=-1  VariantActivationTime=update diagram  EmptyChoice=off  PassThrough=off  PassThroughVariantControl=(default)  EmptyChoiceVariantControl=(default)  AllowFlexibleInterface=on  Latency=0  AutoFrameSizeCalculation=off
  Vectorized Park Transform : Subsystem  mask{no_meas=4}
      mask prompts: no_meas "Number of measurements"
  Vectorized Inverse Park Transform : Subsystem  mask{no_meas=4}
      mask prompts: no_meas "Number of Measurements"
